simulink model "ofdm_txrx_supermimo_coded"  (Simulink 7.7)
settings: Solver=VariableStepDiscrete  SolverMode=SingleTasking  StartTime=0.0  StopTime=2*simOnly_simLength  RelTol=1e-3
PostLoadFcn:
  | ofdm_rx_supermimo_init
InitFcn:
  | ofdm_rx_supermimo_init
StartFcn:
  | ofdm_rx_supermimo_init
values from neighbouring files (symbols this model uses):
  ActionID_AFTransmit = 31    [ofdm_tx_supermimo_init.m]
  ActionID_Disabled = 0    [ofdm_tx_supermimo_init.m]
  ActionID_SetFlagA = 62    [ofdm_tx_supermimo_init.m]
  ActionID_SetFlagB = 61    [ofdm_tx_supermimo_init.m]
  BER_RAM_init_size = 2048    [ofdm_tx_supermimo_init.m]
  channel_h = [1 0; 1 0] * 0.3    [ofdm_tx_supermimo_init.m]
  CPLength = 16    [ofdm_tx_supermimo_init.m]
  longCorr_coef_i = [-4*real(LTS) 0]    [ofdm_rx_supermimo_init.m]
  longCorr_coef_q = [4*imag(LTS) 0]    [ofdm_rx_supermimo_init.m]
  max_CP_length = 16    [ofdm_tx_supermimo_init.m]
  max_num_baseRateSymbols = 31    [ofdm_tx_supermimo_init.m]
  max_num_subcarriers = 64    [ofdm_tx_supermimo_init.m]
  max_num_trainingSymbols = 15    [ofdm_tx_supermimo_init.m]
  max_numBytes = 16384    [ofdm_tx_supermimo_init.m]
  max_OFDM_symbols = 1023    [ofdm_tx_supermimo_init.m]
  modConstellation_qam16 = .75*[1 3 -1 -3]./3    [ofdm_tx_supermimo_init.m]
  modConstellation_qam64 = 0.875*[3 1 5 7 -3 -1 -5 -7]./7    [ofdm_tx_supermimo_init.m]
  modConstellation_qpsk = [1 -1]./sqrt(2)    [ofdm_tx_supermimo_init.m]
  numBaseRateSymbols = numBaseRateSymbols *2    [ofdm_tx_supermimo_init.m]
  numSubcarriers = 64    [ofdm_tx_supermimo_init.m]
  numTrainingSymbols = 2    [ofdm_tx_supermimo_init.m]
  pktTiming_count_payloadStart = pktTiming_count_lsStart + pktTiming_ls_num*pktTiming_ls_length    [ofdm_rx_supermimo_init.m]
  preamble = preamble_scale*[shortsyms_10 longsyms_2]    [ofdm_tx_supermimo_init.m]
  preamble_ROM = preamble_I_ROM + preamble_Q_ROM*2^16    [ofdm_tx_supermimo_init.m]
  RAM_init_size = max_numBytes    (= 16384)    [ofdm_tx_supermimo_init.m]
  RxDataScrambling_Seq = [40 198 78 63 82 173 102 245 48 111 172 115 147 230 216 93 72 65 62 2 205 242 122 90 128 83 105 97 73 10 5 252]    [ofdm_tx_supermimo_init.m]
  shortSymbol_time = shortSymbol_time(1:16).'    [ofdm_tx_supermimo_init.m]
  simOnly_numSamples = length(preamble)+( (numSubcarriers+CPLength)*(numTrainingSymbols + numBaseRateSymbols + numFullRateSymbols/2) )    [ofdm_tx_supermimo_init.m]
  train = [train train]    [ofdm_tx_supermimo_init.m]
  TxDataScrambling_Seq = [40 198 78 63 82 173 102 245 48 111 172 115 147 230 216 93 72 65 62 2 205 242 122 90 128 83 105 97 73 10 5 252]    [ofdm_tx_supermimo_init.m]
undefined symbols (not defined in the file or in its folder): AntA, AntB, BaseRate, Down, gcbh, numBuffs, numBytes, Up, xlBlockLoadCallback, xlGetNormalizedPeriod, xlipgetpartsetting
libraries: xbsIndex_r4 x5430
(part 2 of 49)

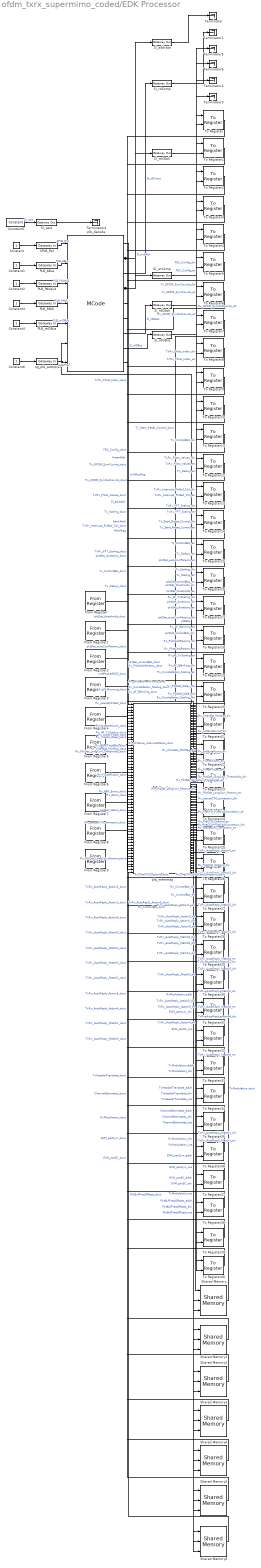
== system ofdm_txrx_supermimo_coded/EDK Processor ==
  Constant{,1..4,6} (6 alike) : Constant
  Constant5 : xbsIndex_r4/Constant  const=0  gui_display_data_type=Fixed-point  arith_type=Unsigned  n_bits=1  bin_pt=0  preci_type=Single  exp_width=8  frac_width=24  explicit_period=on  period=xlGetNormalizedPeriod()  equ=P=C  opselect=C  inp2=PCIN>>17  opr=+  inp1=P  carry=CIN  dbl_ovrd=off  block_type=constant
  From Register : xbsIndex_r4/From Register  shared_memory_name='midPacketRSSI'  init=0  period=xlGetNormalizedPeriod()  ownership=Owned and initialized elsewhere  gui_display_data_type=Fixed-point  arith_type=Unsigned  n_bits=32  bin_pt=0  preci_type=Single  dbl_ovrd=off  block_type=fromreg
      settings: AttributesFormatString=<< %<shared_memory_name> >>  infoedit="Register block that reads data to a shared memory register.  Delay of one sample period."
  From Register1 : xbsIndex_r4/From Register  shared_memory_name='Tx_PktRunning'  init=0  period=xlGetNormalizedPeriod()  ownership=Owned and initialized elsewhere  gui_display_data_type=Fixed-point  arith_type=Unsigned  n_bits=32  bin_pt=0  preci_type=Single  dbl_ovrd=off  block_type=fromreg
      settings: AttributesFormatString=<< %<shared_memory_name> >>  infoedit="Register block that reads data to a shared memory register.  Delay of one sample period."
  From Register2 : xbsIndex_r4/From Register  shared_memory_name='Rx_pktDetEventCount'  init=0  period=xlGetNormalizedPeriod()  ownership=Owned and initialized elsewhere  gui_display_data_type=Fixed-point  arith_type=Unsigned  n_bits=32  bin_pt=0  preci_type=Single  dbl_ovrd=off  block_type=fromreg
      settings: AttributesFormatString=<< %<shared_memory_name> >>  infoedit="Register block that reads data to a shared memory register.  Delay of one sample period."
  From Register3 : xbsIndex_r4/From Register  shared_memory_name='Rx_coarseCFOest'  init=0  period=xlGetNormalizedPeriod()  ownership=Owned and initialized elsewhere  gui_display_data_type=Fixed-point  arith_type=Unsigned  n_bits=32  bin_pt=0  preci_type=Single  dbl_ovrd=off  block_type=fromreg
      settings: AttributesFormatString=<< %<shared_memory_name> >>  infoedit="Register block that reads data to a shared memory register.  Delay of one sample period."
  From Register4 : xbsIndex_r4/From Register  shared_memory_name='Rx_pilotCFOest'  init=0  period=xlGetNormalizedPeriod()  ownership=Owned and initialized elsewhere  gui_display_data_type=Fixed-point  arith_type=Unsigned  n_bits=32  bin_pt=0  preci_type=Single  dbl_ovrd=off  block_type=fromreg
      settings: AttributesFormatString=<< %<shared_memory_name> >>  infoedit="Register block that reads data to a shared memory register.  Delay of one sample period."
  From Register5 : xbsIndex_r4/From Register  shared_memory_name='Rx_pktDone_interruptStatus'  init=0  period=xlGetNormalizedPeriod()  ownership=Owned and initialized elsewhere  gui_display_data_type=Fixed-point  arith_type=Unsigned  n_bits=32  bin_pt=0  preci_type=Single  dbl_ovrd=off  block_type=fromreg
      settings: AttributesFormatString=<< %<shared_memory_name> >>  infoedit="Register block that reads data to a shared memory register.  Delay of one sample period."
  From Register6 : xbsIndex_r4/From Register  shared_memory_name='Rx_BER_TotalBits'  init=0  period=xlGetNormalizedPeriod()  ownership=Owned and initialized elsewhere  gui_display_data_type=Fixed-point  arith_type=Unsigned  n_bits=32  bin_pt=0  preci_type=Single  dbl_ovrd=off  block_type=fromreg
      settings: AttributesFormatString=<< %<shared_memory_name> >>  infoedit="Register block that reads data to a shared memory register.  Delay of one sample period."
  From Register7 : xbsIndex_r4/From Register  shared_memory_name='Rx_BER_Errors'  init=0  period=xlGetNormalizedPeriod()  ownership=Owned and initialized elsewhere  gui_display_data_type=Fixed-point  arith_type=Unsigned  n_bits=32  bin_pt=0  preci_type=Single  dbl_ovrd=off  block_type=fromreg
      settings: AttributesFormatString=<< %<shared_memory_name> >>  infoedit="Register block that reads data to a shared memory register.  Delay of one sample period."
  From Register8 : xbsIndex_r4/From Register  shared_memory_name='Rx_Gains'  init=0  period=xlGetNormalizedPeriod()  ownership=Owned and initialized elsewhere  gui_display_data_type=Fixed-point  arith_type=Unsigned  n_bits=32  bin_pt=0  preci_type=Single  dbl_ovrd=off  block_type=fromreg
      settings: AttributesFormatString=<< %<shared_memory_name> >>  infoedit="Register block that reads data to a shared memory register.  Delay of one sample period."
  From Register9 : xbsIndex_r4/From Register  shared_memory_name='pktDet_status'  init=0  period=xlGetNormalizedPeriod()  ownership=Owned and initialized elsewhere  gui_display_data_type=Fixed-point  arith_type=Unsigned  n_bits=14  bin_pt=0  preci_type=Single  dbl_ovrd=off  block_type=fromreg
      settings: AttributesFormatString=<< %<shared_memory_name> >>  infoedit="Register block that reads data to a shared memory register.  Delay of one sample period."
  PLB_ABus : xbsIndex_r4/Gateway In  gui_display_data_type=Fixed-point  arith_type=Unsigned  n_bits=32  bin_pt=0  preci_type=Single  exp_width=8  frac_width=24  quantization="Round  (unbiased: +/- Inf)"  overflow=Saturate  period=xlGetNormalizedPeriod()  dbl_ovrd=off  timing_constraint=None  locs_specified=off  LOCs={}  inherit_from_input=off  UseAsADC=off  ADCChannel='1'  hdl_port=on  block_type=gatewayin
      settings: infoedit="Gateway in block.  Converts inputs of type Simulink integer, single, double and fixed point to  Xilinx fixed-point or floating-point data type.<br><br>Hardware notes:  In hardware these blocks become top level input ports."  sginterface={'Xilinx'=>{'jtaghwcosim'=>{'non_memory_mapped_port'=>'PLB_ABus'}},'iopad'=>{'constraint'=>'#'}}
  PLB_PAValid : xbsIndex_r4/Gateway In  gui_display_data_type=Fixed-point  arith_type=Unsigned  n_bits=1  bin_pt=0  preci_type=Single  exp_width=8  frac_width=24  quantization="Round  (unbiased: +/- Inf)"  overflow=Saturate  period=xlGetNormalizedPeriod()  dbl_ovrd=off  timing_constraint=None  locs_specified=off  LOCs={}  inherit_from_input=off  UseAsADC=off  ADCChannel='1'  hdl_port=on  block_type=gatewayin
      settings: infoedit="Gateway in block.  Converts inputs of type Simulink integer, single, double and fixed point to  Xilinx fixed-point or floating-point data type.<br><br>Hardware notes:  In hardware these blocks become top level input ports."  sginterface={'Xilinx'=>{'jtaghwcosim'=>{'non_memory_mapped_port'=>'PLB_PAValid'}},'iopad'=>{'constraint'=>'#'}}
  PLB_RNW : xbsIndex_r4/Gateway In  gui_display_data_type=Fixed-point  arith_type=Unsigned  n_bits=1  bin_pt=0  preci_type=Single  exp_width=8  frac_width=24  quantization="Round  (unbiased: +/- Inf)"  overflow=Saturate  period=xlGetNormalizedPeriod()  dbl_ovrd=off  timing_constraint=None  locs_specified=off  LOCs={}  inherit_from_input=off  UseAsADC=off  ADCChannel='1'  hdl_port=on  block_type=gatewayin
      settings: infoedit="Gateway in block.  Converts inputs of type Simulink integer, single, double and fixed point to  Xilinx fixed-point or floating-point data type.<br><br>Hardware notes:  In hardware these blocks become top level input ports."  sginterface={'Xilinx'=>{'jtaghwcosim'=>{'non_memory_mapped_port'=>'PLB_RNW'}},'iopad'=>{'constraint'=>'#'}}
  PLB_wrDBus : xbsIndex_r4/Gateway In  gui_display_data_type=Fixed-point  arith_type=Unsigned  n_bits=32  bin_pt=0  preci_type=Single  exp_width=8  frac_width=24  quantization="Round  (unbiased: +/- Inf)"  overflow=Saturate  period=xlGetNormalizedPeriod()  dbl_ovrd=off  timing_constraint=None  locs_specified=off  LOCs={}  inherit_from_input=off  UseAsADC=off  ADCChannel='1'  hdl_port=on  block_type=gatewayin
      settings: infoedit="Gateway in block.  Converts inputs of type Simulink integer, single, double and fixed point to  Xilinx fixed-point or floating-point data type.<br><br>Hardware notes:  In hardware these blocks become top level input ports."  sginterface={'Xilinx'=>{'jtaghwcosim'=>{'non_memory_mapped_port'=>'PLB_wrDBus'}},'iopad'=>{'constraint'=>'#'}}
  SPLB_Rst : xbsIndex_r4/Gateway In  gui_display_data_type=Fixed-point  arith_type=Unsigned  n_bits=1  bin_pt=0  preci_type=Single  exp_width=8  frac_width=24  quantization="Round  (unbiased: +/- Inf)"  overflow=Saturate  period=xlGetNormalizedPeriod()  dbl_ovrd=off  timing_constraint=None  locs_specified=off  LOCs={}  inherit_from_input=off  UseAsADC=off  ADCChannel='1'  hdl_port=on  block_type=gatewayin
      settings: infoedit="Gateway in block.  Converts inputs of type Simulink integer, single, double and fixed point to  Xilinx fixed-point or floating-point data type.<br><br>Hardware notes:  In hardware these blocks become top level input ports."  sginterface={'Xilinx'=>{'jtaghwcosim'=>{'non_memory_mapped_port'=>'SPLB_Rst'}},'iopad'=>{'constraint'=>'#'}}
  Shared Memory : xbsIndex_r4/Shared Memory  shared_memory_name='TxModulation'  depth=192  ownership=Owned and Initialized Elsewhere  initVector=sin(pi*(0:15)/16)  en=off  mutex=Unprotected  mode=Read and Write  write_mode=Read Before Write  time_out=0  latency=1  explicit_data_type=off  gui_display_data_type=Fixed-point  arith_type=Unsigned  n_bits=4  bin_pt=0  preci_type=Single  implementation=Block RAM  use_rpm=off  block_type=shmem
      settings: AttributesFormatString=<< %<shared_memory_name> >>
  Shared Memory1 : xbsIndex_r4/Shared Memory  shared_memory_name='TxHeaderTranslate'  depth=1024  ownership=Owned and Initialized Elsewhere  initVector=sin(pi*(0:15)/16)  en=off  mutex=Unprotected  mode=Read and Write  write_mode=Read Before Write  time_out=0  latency=1  explicit_data_type=off  gui_display_data_type=Fixed-point  arith_type=Unsigned  n_bits=10  bin_pt=0  preci_type=Single  implementation=Block RAM  use_rpm=off  block_type=shmem
      settings: AttributesFormatString=<< %<shared_memory_name> >>
  Shared Memory2 : xbsIndex_r4/Shared Memory  shared_memory_name='ChannelEstimates'  depth=256  ownership=Owned and Initialized Elsewhere  initVector=sin(pi*(0:15)/16)  en=off  mutex=Unprotected  mode=Read and Write  write_mode=Read Before Write  time_out=0  latency=1  explicit_data_type=off  gui_display_data_type=Fixed-point  arith_type=Unsigned  n_bits=32  bin_pt=0  preci_type=Single  implementation=Block RAM  use_rpm=off  block_type=shmem
      settings: AttributesFormatString=<< %<shared_memory_name> >>
  Shared Memory3 : xbsIndex_r4/Shared Memory  shared_memory_name='RxModulation'  depth=192  ownership=Owned and Initialized Elsewhere  initVector=sin(pi*(0:15)/16)  en=off  mutex=Unprotected  mode=Read and Write  write_mode=Read Before Write  time_out=0  latency=1  explicit_data_type=off  gui_display_data_type=Fixed-point  arith_type=Unsigned  n_bits=4  bin_pt=0  preci_type=Single  implementation=Block RAM  use_rpm=off  block_type=shmem
      settings: AttributesFormatString=<< %<shared_memory_name> >>
  Shared Memory4 : xbsIndex_r4/Shared Memory  shared_memory_name='EVM_perSym'  depth=256  ownership=Owned and Initialized Elsewhere  initVector=sin(pi*(0:15)/16)  en=off  mutex=Unprotected  mode=Read and Write  write_mode=Read Before Write  time_out=0  latency=1  explicit_data_type=off  gui_display_data_type=Fixed-point  arith_type="Signed  (2's comp)"  n_bits=32  bin_pt=14  preci_type=Single  implementation=Block RAM  use_rpm=off  block_type=shmem
      settings: AttributesFormatString=<< %<shared_memory_name> >>
  Shared Memory5 : xbsIndex_r4/Shared Memory  shared_memory_name='EVM_perSC'  depth=64  ownership=Owned and Initialized Elsewhere  initVector=sin(pi*(0:15)/16)  en=off  mutex=Unprotected  mode=Read and Write  write_mode=Read Before Write  time_out=0  latency=1  explicit_data_type=off  gui_display_data_type=Fixed-point  arith_type="Signed  (2's comp)"  n_bits=32  bin_pt=22  preci_type=Single  implementation=Block RAM  use_rpm=off  block_type=shmem
      settings: AttributesFormatString=<< %<shared_memory_name> >>
  Shared Memory6 : xbsIndex_r4/Shared Memory  shared_memory_name='PktBufFreqOffsets'  depth=32  ownership=Owned and Initialized Elsewhere  initVector=sin(pi*(0:15)/16)  en=off  mutex=Unprotected  mode=Read and Write  write_mode=Read Before Write  time_out=0  latency=1  explicit_data_type=off  gui_display_data_type=Fixed-point  arith_type=Unsigned  n_bits=32  bin_pt=32  preci_type=Single  implementation=Block RAM  use_rpm=off  block_type=shmem
      settings: AttributesFormatString=<< %<shared_memory_name> >>
  Sl_addrAck : xbsIndex_r4/Gateway Out  inherit_from_input=off  hdl_port=on  timing_constraint=None  locs_specified=off  LOCs={}  UseAsDAC=off  DACChannel='1'  block_type=gatewayout
      settings: infoedit="Gateway out block.  Converts Xilinx fixed-point or floating-point type inputs into ouputs of type Simulink integer, single, double, or fixed point.<br><br>Hardware notes:  In hardware these blocks become top level output ports or are discarded, depending on how they are configured."  sginterface={'Xilinx'=>{'jtaghwcosim'=>{'non_memory_mapped_port'=>'Sl_addrAck'}},'iopad'=>{'constraint'=>'#'}}
  Sl_rdComp : xbsIndex_r4/Gateway Out  inherit_from_input=off  hdl_port=on  timing_constraint=None  locs_specified=off  LOCs={}  UseAsDAC=off  DACChannel='1'  block_type=gatewayout
      settings: infoedit="Gateway out block.  Converts Xilinx fixed-point or floating-point type inputs into ouputs of type Simulink integer, single, double, or fixed point.<br><br>Hardware notes:  In hardware these blocks become top level output ports or are discarded, depending on how they are configured."  sginterface={'Xilinx'=>{'jtaghwcosim'=>{'non_memory_mapped_port'=>'Sl_rdComp'}},'iopad'=>{'constraint'=>'#'}}
  Sl_rdDAck : xbsIndex_r4/Gateway Out  inherit_from_input=off  hdl_port=on  timing_constraint=None  locs_specified=off  LOCs={}  UseAsDAC=off  DACChannel='1'  block_type=gatewayout
      settings: infoedit="Gateway out block.  Converts Xilinx fixed-point or floating-point type inputs into ouputs of type Simulink integer, single, double, or fixed point.<br><br>Hardware notes:  In hardware these blocks become top level output ports or are discarded, depending on how they are configured."  sginterface={'Xilinx'=>{'jtaghwcosim'=>{'non_memory_mapped_port'=>'Sl_rdDAck'}},'iopad'=>{'constraint'=>'#'}}
  Sl_rdDBus : xbsIndex_r4/Gateway Out  inherit_from_input=off  hdl_port=on  timing_constraint=None  locs_specified=off  LOCs={}  UseAsDAC=off  DACChannel='1'  block_type=gatewayout
      settings: infoedit="Gateway out block.  Converts Xilinx fixed-point or floating-point type inputs into ouputs of type Simulink integer, single, double, or fixed point.<br><br>Hardware notes:  In hardware these blocks become top level output ports or are discarded, depending on how they are configured."  sginterface={'Xilinx'=>{'jtaghwcosim'=>{'non_memory_mapped_port'=>'Sl_rdDBus'}},'iopad'=>{'constraint'=>'#'}}
  Sl_wait : xbsIndex_r4/Gateway Out  inherit_from_input=off  hdl_port=on  timing_constraint=None  locs_specified=off  LOCs={}  UseAsDAC=off  DACChannel='1'  block_type=gatewayout
      settings: infoedit="Gateway out block.  Converts Xilinx fixed-point or floating-point type inputs into ouputs of type Simulink integer, single, double, or fixed point.<br><br>Hardware notes:  In hardware these blocks become top level output ports or are discarded, depending on how they are configured."  sginterface={'Xilinx'=>{'jtaghwcosim'=>{'non_memory_mapped_port'=>'Sl_wait'}},'iopad'=>{'constraint'=>'#'}}
  Sl_wrComp : xbsIndex_r4/Gateway Out  inherit_from_input=off  hdl_port=on  timing_constraint=None  locs_specified=off  LOCs={}  UseAsDAC=off  DACChannel='1'  block_type=gatewayout
      settings: infoedit="Gateway out block.  Converts Xilinx fixed-point or floating-point type inputs into ouputs of type Simulink integer, single, double, or fixed point.<br><br>Hardware notes:  In hardware these blocks become top level output ports or are discarded, depending on how they are configured."  sginterface={'Xilinx'=>{'jtaghwcosim'=>{'non_memory_mapped_port'=>'Sl_wrComp'}},'iopad'=>{'constraint'=>'#'}}
  Sl_wrDAck : xbsIndex_r4/Gateway Out  inherit_from_input=off  hdl_port=on  timing_constraint=None  locs_specified=off  LOCs={}  UseAsDAC=off  DACChannel='1'  block_type=gatewayout
      settings: infoedit="Gateway out block.  Converts Xilinx fixed-point or floating-point type inputs into ouputs of type Simulink integer, single, double, or fixed point.<br><br>Hardware notes:  In hardware these blocks become top level output ports or are discarded, depending on how they are configured."  sginterface={'Xilinx'=>{'jtaghwcosim'=>{'non_memory_mapped_port'=>'Sl_wrDAck'}},'iopad'=>{'constraint'=>'#'}}
  Terminator{,1..6} (7 alike) : Terminator
  To Register : xbsIndex_r4/To Register  shared_memory_name='FEC_Config'  init=0  ownership=Owned and initialized elsewhere  explicit_data_type=on  gui_display_data_type=Fixed-point  arith_type=Unsigned  n_bits=32  bin_pt=0  preci_type=Single  dbl_ovrd=off  block_type=toreg
      settings: AttributesFormatString=<< %<shared_memory_name> >>  infoedit="Register block that writes data to a shared memory register.  Delay of one sample period."
  To Register1 : xbsIndex_r4/To Register  shared_memory_name='Tx_OFDM_SymCounts'  init=0  ownership=Owned and initialized elsewhere  explicit_data_type=on  gui_display_data_type=Fixed-point  arith_type=Unsigned  n_bits=32  bin_pt=0  preci_type=Single  dbl_ovrd=off  block_type=toreg
      settings: AttributesFormatString=<< %<shared_memory_name> >>  infoedit="Register block that writes data to a shared memory register.  Delay of one sample period."
  To Register10 : xbsIndex_r4/To Register  shared_memory_name='Tx_Scaling'  init=0  ownership=Owned and initialized elsewhere  explicit_data_type=on  gui_display_data_type=Fixed-point  arith_type=Unsigned  n_bits=32  bin_pt=0  preci_type=Single  dbl_ovrd=off  block_type=toreg
      settings: AttributesFormatString=<< %<shared_memory_name> >>  infoedit="Register block that writes data to a shared memory register.  Delay of one sample period."
  To Register11 : xbsIndex_r4/To Register  shared_memory_name='pktDet_thresholds'  init=0  ownership=Owned and initialized elsewhere  explicit_data_type=on  gui_display_data_type=Fixed-point  arith_type=Unsigned  n_bits=32  bin_pt=0  preci_type=Single  dbl_ovrd=off  block_type=toreg
      settings: AttributesFormatString=<< %<shared_memory_name> >>  infoedit="Register block that writes data to a shared memory register.  Delay of one sample period."
  To Register12 : xbsIndex_r4/To Register  shared_memory_name='pktDet_durations'  init=0  ownership=Owned and initialized elsewhere  explicit_data_type=on  gui_display_data_type=Fixed-point  arith_type=Unsigned  n_bits=32  bin_pt=0  preci_type=Single  dbl_ovrd=off  block_type=toreg
      settings: AttributesFormatString=<< %<shared_memory_name> >>  infoedit="Register block that writes data to a shared memory register.  Delay of one sample period."
  To Register13 : xbsIndex_r4/To Register  shared_memory_name='pktDet_autoCorrParams'  init=0  ownership=Owned and initialized elsewhere  explicit_data_type=on  gui_display_data_type=Fixed-point  arith_type=Unsigned  n_bits=32  bin_pt=0  preci_type=Single  dbl_ovrd=off  block_type=toreg
      settings: AttributesFormatString=<< %<shared_memory_name> >>  infoedit="Register block that writes data to a shared memory register.  Delay of one sample period."
  To Register14 : xbsIndex_r4/To Register  shared_memory_name='pktDet_controlBits'  init=0  ownership=Owned and initialized elsewhere  explicit_data_type=on  gui_display_data_type=Fixed-point  arith_type=Unsigned  n_bits=32  bin_pt=0  preci_type=Single  dbl_ovrd=off  block_type=toreg
      settings: AttributesFormatString=<< %<shared_memory_name> >>  infoedit="Register block that writes data to a shared memory register.  Delay of one sample period."
  To Register15 : xbsIndex_r4/To Register  shared_memory_name='Rx_AF_TxScaling'  init=0  ownership=Owned and initialized elsewhere  explicit_data_type=on  gui_display_data_type=Fixed-point  arith_type=Unsigned  n_bits=32  bin_pt=0  preci_type=Single  dbl_ovrd=off  block_type=toreg
      settings: AttributesFormatString=<< %<shared_memory_name> >>  infoedit="Register block that writes data to a shared memory register.  Delay of one sample period."
  To Register16 : xbsIndex_r4/To Register  shared_memory_name='Rx_AF_Blanking'  init=0  ownership=Owned and initialized elsewhere  explicit_data_type=on  gui_display_data_type=Fixed-point  arith_type=Unsigned  n_bits=32  bin_pt=0  preci_type=Single  dbl_ovrd=off  block_type=toreg
      settings: AttributesFormatString=<< %<shared_memory_name> >>  infoedit="Register block that writes data to a shared memory register.  Delay of one sample period."
  To Register17 : xbsIndex_r4/To Register  shared_memory_name='Rx_PilotCalcParams'  init=0  ownership=Owned and initialized elsewhere  explicit_data_type=on  gui_display_data_type=Fixed-point  arith_type=Unsigned  n_bits=32  bin_pt=0  preci_type=Single  dbl_ovrd=off  block_type=toreg
      settings: AttributesFormatString=<< %<shared_memory_name> >>  infoedit="Register block that writes data to a shared memory register.  Delay of one sample period."
  To Register18 : xbsIndex_r4/To Register  shared_memory_name='Rx_Constellation_Scaling'  init=0  ownership=Owned and initialized elsewhere  explicit_data_type=on  gui_display_data_type=Fixed-point  arith_type=Unsigned  n_bits=32  bin_pt=0  preci_type=Single  dbl_ovrd=off  block_type=toreg
      settings: AttributesFormatString=<< %<shared_memory_name> >>  infoedit="Register block that writes data to a shared memory register.  Delay of one sample period."
  To Register19 : xbsIndex_r4/To Register  shared_memory_name='Rx_PktDet_Delay'  init=0  ownership=Owned and initialized elsewhere  explicit_data_type=on  gui_display_data_type=Fixed-point  arith_type=Unsigned  n_bits=32  bin_pt=0  preci_type=Single  dbl_ovrd=off  block_type=toreg
      settings: AttributesFormatString=<< %<shared_memory_name> >>  infoedit="Register block that writes data to a shared memory register.  Delay of one sample period."
  To Register2 : xbsIndex_r4/To Register  shared_memory_name='Rx_OFDM_SymbolCounts'  init=0  ownership=Owned and initialized elsewhere  explicit_data_type=on  gui_display_data_type=Fixed-point  arith_type=Unsigned  n_bits=32  bin_pt=0  preci_type=Single  dbl_ovrd=off  block_type=toreg
      settings: AttributesFormatString=<< %<shared_memory_name> >>  infoedit="Register block that writes data to a shared memory register.  Delay of one sample period."
  To Register20 : xbsIndex_r4/To Register  shared_memory_name='Rx_ChanEst_MinMag'  init=0  ownership=Owned and initialized elsewhere  explicit_data_type=on  gui_display_data_type=Fixed-point  arith_type=Unsigned  n_bits=32  bin_pt=0  preci_type=Single  dbl_ovrd=off  block_type=toreg
      settings: AttributesFormatString=<< %<shared_memory_name> >>  infoedit="Register block that writes data to a shared memory register.  Delay of one sample period."
  To Register21 : xbsIndex_r4/To Register  shared_memory_name='Rx_pktByteNums'  init=0  ownership=Owned and initialized elsewhere  explicit_data_type=on  gui_display_data_type=Fixed-point  arith_type=Unsigned  n_bits=32  bin_pt=0  preci_type=Single  dbl_ovrd=off  block_type=toreg
      settings: AttributesFormatString=<< %<shared_memory_name> >>  infoedit="Register block that writes data to a shared memory register.  Delay of one sample period."
  To Register22 : xbsIndex_r4/To Register  shared_memory_name='Rx_FixedPktLen'  init=0  ownership=Owned and initialized elsewhere  explicit_data_type=on  gui_display_data_type=Fixed-point  arith_type=Unsigned  n_bits=32  bin_pt=0  preci_type=Single  dbl_ovrd=off  block_type=toreg
      settings: AttributesFormatString=<< %<shared_memory_name> >>  infoedit="Register block that writes data to a shared memory register.  Delay of one sample period."
  To Register23 : xbsIndex_r4/To Register  shared_memory_name='Rx_PktDet_LongCorr_Thresholds'  init=0  ownership=Owned and initialized elsewhere  explicit_data_type=on  gui_display_data_type=Fixed-point  arith_type=Unsigned  n_bits=32  bin_pt=0  preci_type=Single  dbl_ovrd=off  block_type=toreg
      settings: AttributesFormatString=<< %<shared_memory_name> >>  infoedit="Register block that writes data to a shared memory register.  Delay of one sample period."
  To Register24 : xbsIndex_r4/To Register  shared_memory_name='Rx_PktDet_LongCorr_Params'  init=0  ownership=Owned and initialized elsewhere  explicit_data_type=on  gui_display_data_type=Fixed-point  arith_type=Unsigned  n_bits=32  bin_pt=0  preci_type=Single  dbl_ovrd=off  block_type=toreg
      settings: AttributesFormatString=<< %<shared_memory_name> >>  infoedit="Register block that writes data to a shared memory register.  Delay of one sample period."
  To Register25 : xbsIndex_r4/To Register  shared_memory_name='Rx_coarseCFO_correction'  init=0  ownership=Owned and initialized elsewhere  explicit_data_type=on  gui_display_data_type=Fixed-point  arith_type=Unsigned  n_bits=32  bin_pt=0  preci_type=Single  dbl_ovrd=off  block_type=toreg
      settings: AttributesFormatString=<< %<shared_memory_name> >>  infoedit="Register block that writes data to a shared memory register.  Delay of one sample period."
  To Register26 : xbsIndex_r4/To Register  shared_memory_name='Rx_PreCFO_PilotCalcCorrection'  init=0  ownership=Owned and initialized elsewhere  explicit_data_type=on  gui_display_data_type=Fixed-point  arith_type=Unsigned  n_bits=32  bin_pt=0  preci_type=Single  dbl_ovrd=off  block_type=toreg
      settings: AttributesFormatString=<< %<shared_memory_name> >>  infoedit="Register block that writes data to a shared memory register.  Delay of one sample period."
  To Register27 : xbsIndex_r4/To Register  shared_memory_name='Rx_PreCFO_Options'  init=0  ownership=Owned and initialized elsewhere  explicit_data_type=on  gui_display_data_type=Fixed-point  arith_type=Unsigned  n_bits=32  bin_pt=0  preci_type=Single  dbl_ovrd=off  block_type=toreg
      settings: AttributesFormatString=<< %<shared_memory_name> >>  infoedit="Register block that writes data to a shared memory register.  Delay of one sample period."
  To Register28 : xbsIndex_r4/To Register  shared_memory_name='Rx_ControlBits'  init=0  ownership=Owned and initialized elsewhere  explicit_data_type=on  gui_display_data_type=Fixed-point  arith_type=Unsigned  n_bits=32  bin_pt=0  preci_type=Single  dbl_ovrd=off  block_type=toreg
      settings: AttributesFormatString=<< %<shared_memory_name> >>  infoedit="Register block that writes data to a shared memory register.  Delay of one sample period."
  To Register29 : xbsIndex_r4/To Register  shared_memory_name='TxRx_AutoReply_Action3'  init=0  ownership=Owned and initialized elsewhere  explicit_data_type=on  gui_display_data_type=Fixed-point  arith_type=Unsigned  n_bits=32  bin_pt=0  preci_type=Single  dbl_ovrd=off  block_type=toreg
      settings: AttributesFormatString=<< %<shared_memory_name> >>  infoedit="Register block that writes data to a shared memory register.  Delay of one sample period."
  To Register3 : xbsIndex_r4/To Register  shared_memory_name='TxRx_Pilots_Values'  init=0  ownership=Owned and initialized elsewhere  explicit_data_type=on  gui_display_data_type=Fixed-point  arith_type=Unsigned  n_bits=32  bin_pt=0  preci_type=Single  dbl_ovrd=off  block_type=toreg
      settings: AttributesFormatString=<< %<shared_memory_name> >>  infoedit="Register block that writes data to a shared memory register.  Delay of one sample period."
  To Register30 : xbsIndex_r4/To Register  shared_memory_name='TxRx_AutoReply_Action2'  init=0  ownership=Owned and initialized elsewhere  explicit_data_type=on  gui_display_data_type=Fixed-point  arith_type=Unsigned  n_bits=32  bin_pt=0  preci_type=Single  dbl_ovrd=off  block_type=toreg
      settings: AttributesFormatString=<< %<shared_memory_name> >>  infoedit="Register block that writes data to a shared memory register.  Delay of one sample period."
  To Register31 : xbsIndex_r4/To Register  shared_memory_name='TxRx_AutoReply_Action1'  init=0  ownership=Owned and initialized elsewhere  explicit_data_type=on  gui_display_data_type=Fixed-point  arith_type=Unsigned  n_bits=32  bin_pt=0  preci_type=Single  dbl_ovrd=off  block_type=toreg
      settings: AttributesFormatString=<< %<shared_memory_name> >>  infoedit="Register block that writes data to a shared memory register.  Delay of one sample period."
  To Register32 : xbsIndex_r4/To Register  shared_memory_name='TxRx_AutoReply_Action0'  init=0  ownership=Owned and initialized elsewhere  explicit_data_type=on  gui_display_data_type=Fixed-point  arith_type=Unsigned  n_bits=32  bin_pt=0  preci_type=Single  dbl_ovrd=off  block_type=toreg
      settings: AttributesFormatString=<< %<shared_memory_name> >>  infoedit="Register block that writes data to a shared memory register.  Delay of one sample period."
  To Register33 : xbsIndex_r4/To Register  shared_memory_name='TxRx_AutoReply_Match5'  init=0  ownership=Owned and initialized elsewhere  explicit_data_type=on  gui_display_data_type=Fixed-point  arith_type=Unsigned  n_bits=32  bin_pt=0  preci_type=Single  dbl_ovrd=off  block_type=toreg
      settings: AttributesFormatString=<< %<shared_memory_name> >>  infoedit="Register block that writes data to a shared memory register.  Delay of one sample period."
  To Register34 : xbsIndex_r4/To Register  shared_memory_name='TxRx_AutoReply_Match4'  init=0  ownership=Owned and initialized elsewhere  explicit_data_type=on  gui_display_data_type=Fixed-point  arith_type=Unsigned  n_bits=32  bin_pt=0  preci_type=Single  dbl_ovrd=off  block_type=toreg
      settings: AttributesFormatString=<< %<shared_memory_name> >>  infoedit="Register block that writes data to a shared memory register.  Delay of one sample period."
  To Register35 : xbsIndex_r4/To Register  shared_memory_name='TxRx_AutoReply_Match3'  init=0  ownership=Owned and initialized elsewhere  explicit_data_type=on  gui_display_data_type=Fixed-point  arith_type=Unsigned  n_bits=32  bin_pt=0  preci_type=Single  dbl_ovrd=off  block_type=toreg
      settings: AttributesFormatString=<< %<shared_memory_name> >>  infoedit="Register block that writes data to a shared memory register.  Delay of one sample period."
  To Register36 : xbsIndex_r4/To Register  shared_memory_name='TxRx_AutoReply_Match2'  init=0  ownership=Owned and initialized elsewhere  explicit_data_type=on  gui_display_data_type=Fixed-point  arith_type=Unsigned  n_bits=32  bin_pt=0  preci_type=Single  dbl_ovrd=off  block_type=toreg
      settings: AttributesFormatString=<< %<shared_memory_name> >>  infoedit="Register block that writes data to a shared memory register.  Delay of one sample period."
  To Register37 : xbsIndex_r4/To Register  shared_memory_name='TxRx_AutoReply_Action5'  init=0  ownership=Owned and initialized elsewhere  explicit_data_type=on  gui_display_data_type=Fixed-point  arith_type=Unsigned  n_bits=32  bin_pt=0  preci_type=Single  dbl_ovrd=off  block_type=toreg
      settings: AttributesFormatString=<< %<shared_memory_name> >>  infoedit="Register block that writes data to a shared memory register.  Delay of one sample period."
  To Register38 : xbsIndex_r4/To Register  shared_memory_name='TxRx_AutoReply_Action4'  init=0  ownership=Owned and initialized elsewhere  explicit_data_type=on  gui_display_data_type=Fixed-point  arith_type=Unsigned  n_bits=32  bin_pt=0  preci_type=Single  dbl_ovrd=off  block_type=toreg
      settings: AttributesFormatString=<< %<shared_memory_name> >>  infoedit="Register block that writes data to a shared memory register.  Delay of one sample period."
  To Register39 : xbsIndex_r4/To Register  shared_memory_name='TxRx_AutoReply_Match1'  init=0  ownership=Owned and initialized elsewhere  explicit_data_type=on  gui_display_data_type=Fixed-point  arith_type=Unsigned  n_bits=32  bin_pt=0  preci_type=Single  dbl_ovrd=off  block_type=toreg
      settings: AttributesFormatString=<< %<shared_memory_name> >>  infoedit="Register block that writes data to a shared memory register.  Delay of one sample period."
  To Register4 : xbsIndex_r4/To Register  shared_memory_name='TxRx_Pilots_Index'  init=0  ownership=Owned and initialized elsewhere  explicit_data_type=on  gui_display_data_type=Fixed-point  arith_type=Unsigned  n_bits=32  bin_pt=0  preci_type=Single  dbl_ovrd=off  block_type=toreg
      settings: AttributesFormatString=<< %<shared_memory_name> >>  infoedit="Register block that writes data to a shared memory register.  Delay of one sample period."
  To Register40 : xbsIndex_r4/To Register  shared_memory_name='TxRx_AutoReply_Match0'  init=0  ownership=Owned and initialized elsewhere  explicit_data_type=on  gui_display_data_type=Fixed-point  arith_type=Unsigned  n_bits=32  bin_pt=0  preci_type=Single  dbl_ovrd=off  block_type=toreg
      settings: AttributesFormatString=<< %<shared_memory_name> >>  infoedit="Register block that writes data to a shared memory register.  Delay of one sample period."
  To Register5 : xbsIndex_r4/To Register  shared_memory_name='TxRx_Interrupt_PktBuf_Ctrl'  init=0  ownership=Owned and initialized elsewhere  explicit_data_type=on  gui_display_data_type=Fixed-point  arith_type=Unsigned  n_bits=32  bin_pt=0  preci_type=Single  dbl_ovrd=off  block_type=toreg
      settings: AttributesFormatString=<< %<shared_memory_name> >>  infoedit="Register block that writes data to a shared memory register.  Delay of one sample period."
  To Register6 : xbsIndex_r4/To Register  shared_memory_name='TxRx_FFT_Scaling'  init=0  ownership=Owned and initialized elsewhere  explicit_data_type=on  gui_display_data_type=Fixed-point  arith_type=Unsigned  n_bits=12  bin_pt=0  preci_type=Single  dbl_ovrd=off  block_type=toreg
      settings: AttributesFormatString=<< %<shared_memory_name> >>  infoedit="Register block that writes data to a shared memory register.  Delay of one sample period."
  To Register7 : xbsIndex_r4/To Register  shared_memory_name='Tx_Start_Reset_Control'  init=0  ownership=Owned and initialized elsewhere  explicit_data_type=on  gui_display_data_type=Fixed-point  arith_type=Unsigned  n_bits=32  bin_pt=0  preci_type=Single  dbl_ovrd=off  block_type=toreg
      settings: AttributesFormatString=<< %<shared_memory_name> >>  infoedit="Register block that writes data to a shared memory register.  Delay of one sample period."
  To Register8 : xbsIndex_r4/To Register  shared_memory_name='Tx_ControlBits'  init=0  ownership=Owned and initialized elsewhere  explicit_data_type=on  gui_display_data_type=Fixed-point  arith_type=Unsigned  n_bits=32  bin_pt=0  preci_type=Single  dbl_ovrd=off  block_type=toreg
      settings: AttributesFormatString=<< %<shared_memory_name> >>  infoedit="Register block that writes data to a shared memory register.  Delay of one sample period."
  To Register9 : xbsIndex_r4/To Register  shared_memory_name='Tx_Delays'  init=0  ownership=Owned and initialized elsewhere  explicit_data_type=on  gui_display_data_type=Fixed-point  arith_type=Unsigned  n_bits=32  bin_pt=0  preci_type=Single  dbl_ovrd=off  block_type=toreg
      settings: AttributesFormatString=<< %<shared_memory_name> >>  infoedit="Register block that writes data to a shared memory register.  Delay of one sample period."
  plb_decode : xbsIndex_r4/MCode  mfname=xlmax  explicit_period=off  period=1  dbl_ovrd=off  enable_stdout=off  enable_debug=off  mfilecontent=|  suppress_output=on  defparams={}  hide_port_list={}  block_type=mcode
      | function [wrDBusReg, addrAck, rdComp, wrDAck, bankAddr, RNWReg, rdDAck, rdDBus, linearAddr] = plb_bus_decode(plbRst, plbABus, plbPAValid, plbRNW, plbWrDBus, rdData, addrPref)
      | 
      | % constant variables (TODO: should pass from outside)
      | ADDRPREF_LEN = 16;
      | BANKADDR_LEN = 2;
      | LINEARADDR_LEN = 12;
      | ABUS_LEN = 32;
      | DBUS_LEN = 32;
      | 
      | % declare and initialize persistent variables
      | % register input bus signals
      | persistent plbRstReg_, plbRstReg_ = xl_state(0, {xlBoolean});
      | persistent plbABusReg_, plbABusReg_ = xl_state(0, {xlUnsigned, ABUS_LEN, 0});
      | persistent plbPAValidReg_, plbPAValidReg_ = xl_state(0, {xlBoolean});
      | persistent plbRNWReg_, plbRNWReg_ = xl_state(0, {xlUnsigned, 1, 0});
      | persistent plbWrDBusReg_, plbWrDBusReg_ = xl_state(0, {xlUnsigned, DBUS_LEN, 0});
      | 
      | % ===== rest of the outputs =====
      | 
      | bankAddr   = xl_slice(plbABusReg_, 2+BANKADDR_LEN+LINEARADDR_LEN-1, 2+LINEARADDR_LEN);
      | linearAddr = xl_slice(plbABusReg_, 2+LINEARADDR_LEN-1, 2);
      | RNWReg = plbRNWReg_;
      | wrDBusReg = plbWrDBusReg_;
      | 
      | % ===== p_select =====
      | 
      | % register PAValid
      | persistent aValidReg, aValidReg = xl_state(0, {xlBoolean});
      | aValidReg = plbPAValidReg_;
      | 
      | % extract and register the address prefix
      | addrPref_in = xl_slice(plbABusReg_, xl_nbits(plbABusReg_)-1, xl_nbits(plbABusReg_)-ADDRPREF_LEN);
      | if addrPref_in == addrPref
      |     ps1 = true;
      | else 
      |     ps1 = false;
      | end 
      | 
      | persistent ps1Reg, ps1Reg = xl_state(0, ps1);
      | ps1Reg = ps1;
      | 
      | ps = xl_and(ps1Reg, aValidReg);
      | 
      | % ===== addrAck =====
      | 
      | % register ps
      | persistent psReg, psReg = xl_state(0, ps);
      | 
      | addrAck = xfix({xlUnsigned, 1, 0}, xl_and(xl_not(plbRstReg_), ps, xl_not(psReg)));
      | 
      | psReg = ps;
      | 
      | % ===== rdComp, rd/wr DAck =====
      |  
      | rdComp1 = xfix({xlUnsigned, 1, 0}, xl_and(addrAck, RNWReg));
      | 
      | NUM_rdCompDelay = 3;
      | persistent rdCompDelay, rdCompDelay = xl_state(zeros(1, NUM_rdCompDelay), rdComp1, NUM_rdCompDelay);
      | rdComp2 = rdCompDelay.back;
      | rdCompDelay.push_front_pop_back(rdComp1);
      | 
      | persistent rdCompReg, rdCompReg = xl_state(0, rdComp1);
      | rdComp = rdCompReg;
      | rdCompReg = rdComp2;
      | 
      | persistent rdDAckReg, rdDAckReg = xl_state(0, rdComp1);
      | rdDAck = rdDAckReg;
      | rdDAckReg = rdComp;
      | 
      | persistent wrDAckReg, wrDAckReg = xl_state(0, addrAck);
      | wrDAck = wrDAckReg;
      | wrDAckReg = xl_and(addrAck, xl_not(RNWReg));
      | 
      | % ===== rdDBus =====
      | 
      | rdSel = xl_or(rdComp2, rdComp);
      | 
      | if rdSel == 1
      |     rdDBus1 = rdData;
      | else
      |     rdDBus1 = 0;
      | end % if
      | 
      | persistent rdDBusReg, rdDBusReg = xl_state(0, rdDBus1);
      | rdDBus = rdDBusReg;
      | rdDBusReg = rdDBus1;
      | 
      | % rdDBus = xl_concat(rdDBus32, rdDBus32);
      | % rdDBus = rdDBus32;
      | 
      | % ===== update the persistent variables =====
      | 
      | plbRstReg_ = plbRst;
      | plbABusReg_ = plbABus;
      | plbPAValidReg_ = plbPAValid;
      | plbRNWReg_ = plbRNW;
      | plbWrDBusReg_ = xl_slice(plbWrDBus, DBUS_LEN-1, 0);
  plb_memmap : xbsIndex_r4/MCode  mfname=xlmax  explicit_period=off  period=1  dbl_ovrd=off  enable_stdout=off  enable_debug=off  mfilecontent=|  suppress_output=on  defparams={}  hide_port_list={}  block_type=mcode
      | function [read_bank_out, sm_FEC_Config_din, sm_FEC_Config_en, sm_Tx_OFDM_SymCounts_din, sm_Tx_OFDM_SymCounts_en, sm_Rx_OFDM_SymbolCounts_din, sm_Rx_OFDM_SymbolCounts_en, sm_TxRx_Pilots_Values_din, sm_TxRx_Pilots_Values_en, sm_TxRx_Pilots_Index_din, sm_TxRx_Pilots_Index_en, sm_TxRx_Interrupt_PktBuf_Ctrl_din, sm_TxRx_Interrupt_PktBuf_Ctrl_en, sm_TxRx_FFT_Scaling_din, sm_TxRx_FFT_Scaling_en, sm_Tx_Start_Reset_Control_din, sm_Tx_Start_Reset_Control_en, sm_Tx_ControlBits_din, sm_Tx_ControlBits_en, sm_Tx_Delays_din, sm_Tx_Delays_en, sm_Tx_Scaling_din, sm_Tx_Scaling_en, sm_pktDet_thresholds_din, sm_pktDet_thresholds_en, sm_pktDet_durations_din, sm_pktDet_durations_en, sm_pktDet_autoCorrParams_din, sm_pktDet_autoCorrParams_en, sm_pktDet_controlBits_din, sm_pktDet_controlBits_en, sm_Rx_AF_TxScaling_din, sm_Rx_AF_TxScaling_en, sm_Rx_AF_Blanking_din, sm_Rx_AF_Blanking_en, sm_Rx_PilotCalcParams_din, sm_Rx_PilotCalcParams_en, sm_Rx_Constellation_Scaling_din, sm_Rx_Constellation_Scaling_en, sm_Rx_PktDet_Delay_din, sm_Rx_PktDet_Delay_en, sm_Rx_ChanEst_MinMag_din, sm_Rx_ChanEst_MinMag_en, sm_Rx_pktByteNums_din, sm_Rx_pktByteNums_en, sm_Rx_FixedPktLen_din, sm_Rx_FixedPktLen_en, sm_Rx_PktDet_LongCorr_Thresholds_din, sm_Rx_PktDet_LongCorr_Thresholds_en, sm_Rx_PktDet_LongCorr_Params_din, sm_Rx_PktDet_LongCorr_Params_en, sm_Rx_coarseCFO_correction_din, sm_Rx_coarseCFO_correction_en, sm_Rx_PreCFO_PilotCalcCorrection_din, sm_Rx_PreCFO_PilotCalcCorrection_en, sm_Rx_PreCFO_Options_din, sm_Rx_PreCFO_Options_en, sm_Rx_ControlBits_din, sm_Rx_ControlBits_en, sm_TxRx_AutoReply_Action3_din, sm_TxRx_AutoReply_Action3_en, sm_TxRx_AutoReply_Action2_din, sm_TxRx_AutoReply_Action2_en, sm_TxRx_AutoReply_Action1_din, sm_TxRx_AutoReply_Action1_en, sm_TxRx_AutoReply_Action0_din, sm_TxRx_AutoReply_Action0_en, sm_TxRx_AutoReply_Match5_din, sm_TxRx_AutoReply_Match5_en, sm_TxRx_AutoReply_Match4_din, sm_TxRx_AutoReply_Match4_en, sm_TxRx_AutoReply_Match3_din, sm_TxRx_AutoReply_Match3_en, sm_TxRx_AutoReply_Match2_din, sm_TxRx_AutoReply_Match2_en, sm_TxRx_AutoReply_Action5_din, sm_TxRx_AutoReply_Action5_en, sm_TxRx_AutoReply_Action4_din, sm_TxRx_AutoReply_Action4_en, sm_TxRx_AutoReply_Match1_din, sm_TxRx_AutoReply_Match1_en, sm_TxRx_AutoReply_Match0_din, sm_TxRx_AutoReply_Match0_en, sm_TxModulation_addr, sm_TxModulation_din, sm_TxModulation_we, sm_TxHeaderTranslate_addr, sm_TxHeaderTranslate_din, sm_TxHeaderTranslate_we, sm_ChannelEstimates_addr, sm_ChannelEstimates_din, sm_ChannelEstimates_we, sm_RxModulation_addr, sm_RxModulation_din, sm_RxModulation_we, sm_EVM_perSym_addr, sm_EVM_perSym_din, sm_EVM_perSym_we, sm_EVM_perSC_addr, sm_EVM_perSC_din, sm_EVM_perSC_we, sm_PktBufFreqOffsets_addr, sm_PktBufFreqOffsets_din, sm_PktBufFreqOffsets_we] = plb_memmap(wrDBus, bankAddr, linearAddr, RNWReg, addrAck, sm_midPacketRSSI, sm_Tx_PktRunning, sm_Rx_pktDetEventCount, sm_Rx_coarseCFOest, sm_Rx_pilotCFOest, sm_Rx_pktDone_interruptStatus, sm_Rx_BER_TotalBits, sm_Rx_BER_Errors, sm_Rx_Gains, sm_pktDet_status, sm_FEC_Config, sm_Tx_OFDM_SymCounts, sm_Rx_OFDM_SymbolCounts, sm_TxRx_Pilots_Values, sm_TxRx_Pilots_Index, sm_TxRx_Interrupt_PktBuf_Ctrl, sm_TxRx_FFT_Scaling, sm_Tx_Start_Reset_Control, sm_Tx_ControlBits, sm_Tx_Delays, sm_Tx_Scaling, sm_pktDet_thresholds, sm_pktDet_durations, sm_pktDet_autoCorrParams, sm_pktDet_controlBits, sm_Rx_AF_TxScaling, sm_Rx_AF_Blanking, sm_Rx_PilotCalcParams, sm_Rx_Constellation_Scaling, sm_Rx_PktDet_Delay, sm_Rx_ChanEst_MinMag, sm_Rx_pktByteNums, sm_Rx_FixedPktLen, sm_Rx_PktDet_LongCorr_Thresholds, sm_Rx_PktDet_LongCorr_Params, sm_Rx_coarseCFO_correction, sm_Rx_PreCFO_PilotCalcCorrection, sm_Rx_PreCFO_Options, sm_Rx_ControlBits, sm_TxRx_AutoReply_Action3, sm_TxRx_AutoReply_Action2, sm_TxRx_AutoReply_Action1, sm_TxRx_AutoReply_Action0, sm_TxRx_AutoReply_Match5, sm_TxRx_AutoReply_Match4, sm_TxRx_AutoReply_Match3, sm_TxRx_AutoReply_Match2, sm_TxRx_AutoReply_Action5, sm_TxRx_AutoReply_Action4, sm_TxRx_AutoReply_Match1, sm_TxRx_AutoReply_Match0, sm_TxModulation, sm_TxHeaderTranslate, sm_ChannelEstimates, sm_RxModulation, sm_EVM_perSym, sm_EVM_perSC, sm_PktBufFreqOffsets)
      | 
      | 
      | % connvert the input data to UFix_32_0 (the bus data type)
      | % 'From Register' blocks
      | % sm_midPacketRSSI_bus = xfix({xlUnsigned, 32, 0}, 0);
      | sm_midPacketRSSI_bus = xl_force(sm_midPacketRSSI, xlUnsigned, 0);
      | 
      | % sm_Tx_PktRunning_bus = xfix({xlUnsigned, 32, 0}, 0);
      | sm_Tx_PktRunning_bus = xl_force(sm_Tx_PktRunning, xlUnsigned, 0);
      | 
      | % sm_Rx_pktDetEventCount_bus = xfix({xlUnsigned, 32, 0}, 0);
      | sm_Rx_pktDetEventCount_bus = xl_force(sm_Rx_pktDetEventCount, xlUnsigned, 0);
      | 
      | % sm_Rx_coarseCFOest_bus = xfix({xlUnsigned, 32, 0}, 0);
      | sm_Rx_coarseCFOest_bus = xl_force(sm_Rx_coarseCFOest, xlUnsigned, 0);
      | 
      | % sm_Rx_pilotCFOest_bus = xfix({xlUnsigned, 32, 0}, 0);
      | sm_Rx_pilotCFOest_bus = xl_force(sm_Rx_pilotCFOest, xlUnsigned, 0);
      | 
      | % sm_Rx_pktDone_interruptStatus_bus = xfix({xlUnsigned, 32, 0}, 0);
      | sm_Rx_pktDone_interruptStatus_bus = xl_force(sm_Rx_pktDone_interruptStatus, xlUnsigned, 0);
      | 
      | % sm_Rx_BER_TotalBits_bus = xfix({xlUnsigned, 32, 0}, 0);
      | sm_Rx_BER_TotalBits_bus = xl_force(sm_Rx_BER_TotalBits, xlUnsigned, 0);
      | 
      | % sm_Rx_BER_Errors_bus = xfix({xlUnsigned, 32, 0}, 0);
      | sm_Rx_BER_Errors_bus = xl_force(sm_Rx_BER_Errors, xlUnsigned, 0);
      | 
      | % sm_Rx_Gains_bus = xfix({xlUnsigned, 32, 0}, 0);
      | sm_Rx_Gains_bus = xl_force(sm_Rx_Gains, xlUnsigned, 0);
      | 
      | % sm_pktDet_status_bus = xfix({xlUnsigned, 32, 0}, 0);
      | sm_pktDet_status_bus = xl_force(sm_pktDet_status, xlUnsigned, 0);
      | 
      | % 'To Register' blocks
      | 
      | % sm_FEC_Config_dout = xfix({xlUnsigned, 32, 0}, 0);
      | sm_FEC_Config_dout = xl_force(sm_FEC_Config, xlUnsigned, 0);
      | 
      | % sm_Tx_OFDM_SymCounts_dout = xfix({xlUnsigned, 32, 0}, 0);
      | sm_Tx_OFDM_SymCounts_dout = xl_force(sm_Tx_OFDM_SymCounts, xlUnsigned, 0);
      | 
      | % sm_Rx_OFDM_SymbolCounts_dout = xfix({xlUnsigned, 32, 0}, 0);
      | sm_Rx_OFDM_SymbolCounts_dout = xl_force(sm_Rx_OFDM_SymbolCounts, xlUnsigned, 0);
      | 
      | % sm_TxRx_Pilots_Values_dout = xfix({xlUnsigned, 32, 0}, 0);
      | sm_TxRx_Pilots_Values_dout = xl_force(sm_TxRx_Pilots_Values, xlUnsigned, 0);
      | 
      | % sm_TxRx_Pilots_Index_dout = xfix({xlUnsigned, 32, 0}, 0);
      | sm_TxRx_Pilots_Index_dout = xl_force(sm_TxRx_Pilots_Index, xlUnsigned, 0);
      | 
      | % sm_TxRx_Interrupt_PktBuf_Ctrl_dout = xfix({xlUnsigned, 32, 0}, 0);
      | sm_TxRx_Interrupt_PktBuf_Ctrl_dout = xl_force(sm_TxRx_Interrupt_PktBuf_Ctrl, xlUnsigned, 0);
      | 
      | % sm_TxRx_FFT_Scaling_dout = xfix({xlUnsigned, 32, 0}, 0);
      | sm_TxRx_FFT_Scaling_dout = xl_force(sm_TxRx_FFT_Scaling, xlUnsigned, 0);
      | 
      | % sm_Tx_Start_Reset_Control_dout = xfix({xlUnsigned, 32, 0}, 0);
      | sm_Tx_Start_Reset_Control_dout = xl_force(sm_Tx_Start_Reset_Control, xlUnsigned, 0);
      | 
      | % sm_Tx_ControlBits_dout = xfix({xlUnsigned, 32, 0}, 0);
      | sm_Tx_ControlBits_dout = xl_force(sm_Tx_ControlBits, xlUnsigned, 0);
      | 
      | % sm_Tx_Delays_dout = xfix({xlUnsigned, 32, 0}, 0);
      | sm_Tx_Delays_dout = xl_force(sm_Tx_Delays, xlUnsigned, 0);
      | 
      | % sm_Tx_Scaling_dout = xfix({xlUnsigned, 32, 0}, 0);
      | sm_Tx_Scaling_dout = xl_force(sm_Tx_Scaling, xlUnsigned, 0);
      | 
      | % sm_pktDet_thresholds_dout = xfix({xlUnsigned, 32, 0}, 0);
      | sm_pktDet_thresholds_dout = xl_force(sm_pktDet_thresholds, xlUnsigned, 0);
      | 
      | % sm_pktDet_durations_dout = xfix({xlUnsigned, 32, 0}, 0);
      | sm_pktDet_durations_dout = xl_force(sm_pktDet_durations, xlUnsigned, 0);
      | 
      | % sm_pktDet_autoCorrParams_dout = xfix({xlUnsigned, 32, 0}, 0);
      | sm_pktDet_autoCorrParams_dout = xl_force(sm_pktDet_autoCorrParams, xlUnsigned, 0);
      | 
      | % sm_pktDet_controlBits_dout = xfix({xlUnsigned, 32, 0}, 0);
      | sm_pktDet_controlBits_dout = xl_force(sm_pktDet_controlBits, xlUnsigned, 0);
      | 
      | % sm_Rx_AF_TxScaling_dout = xfix({xlUnsigned, 32, 0}, 0);
      | sm_Rx_AF_TxScaling_dout = xl_force(sm_Rx_AF_TxScaling, xlUnsigned, 0);
      | 
      | % sm_Rx_AF_Blanking_dout = xfix({xlUnsigned, 32, 0}, 0);
      | sm_Rx_AF_Blanking_dout = xl_force(sm_Rx_AF_Blanking, xlUnsigned, 0);
      | 
      | % sm_Rx_PilotCalcParams_dout = xfix({xlUnsigned, 32, 0}, 0);
      | sm_Rx_PilotCalcParams_dout = xl_force(sm_Rx_PilotCalcParams, xlUnsigned, 0);
      | 
      | % sm_Rx_Constellation_Scaling_dout = xfix({xlUnsigned, 32, 0}, 0);
      | sm_Rx_Constellation_Scaling_dout = xl_force(sm_Rx_Constellation_Scaling, xlUnsigned, 0);
      | 
      | % sm_Rx_PktDet_Delay_dout = xfix({xlUnsigned, 32, 0}, 0);
      | sm_Rx_PktDet_Delay_dout = xl_force(sm_Rx_PktDet_Delay, xlUnsigned, 0);
      | 
      | % sm_Rx_ChanEst_MinMag_dout = xfix({xlUnsigned, 32, 0}, 0);
      | sm_Rx_ChanEst_MinMag_dout = xl_force(sm_Rx_ChanEst_MinMag, xlUnsigned, 0);
      | 
      | % sm_Rx_pktByteNums_dout = xfix({xlUnsigned, 32, 0}, 0);
      | sm_Rx_pktByteNums_dout = xl_force(sm_Rx_pktByteNums, xlUnsigned, 0);
      | 
      | % sm_Rx_FixedPktLen_dout = xfix({xlUnsigned, 32, 0}, 0);
      | sm_Rx_FixedPktLen_dout = xl_force(sm_Rx_FixedPktLen, xlUnsigned, 0);
      | 
      | % sm_Rx_PktDet_LongCorr_Thresholds_dout = xfix({xlUnsigned, 32, 0}, 0);
      | sm_Rx_PktDet_LongCorr_Thresholds_dout = xl_force(sm_Rx_PktDet_LongCorr_Thresholds, xlUnsigned, 0);
      | 
      | % sm_Rx_PktDet_LongCorr_Params_dout = xfix({xlUnsigned, 32, 0}, 0);
      | sm_Rx_PktDet_LongCorr_Params_dout = xl_force(sm_Rx_PktDet_LongCorr_Params, xlUnsigned, 0);
      | 
      | % sm_Rx_coarseCFO_correction_dout = xfix({xlUnsigned, 32, 0}, 0);
      | sm_Rx_coarseCFO_correction_dout = xl_force(sm_Rx_coarseCFO_correction, xlUnsigned, 0);
      | 
      | % sm_Rx_PreCFO_PilotCalcCorrection_dout = xfix({xlUnsigned, 32, 0}, 0);
      | sm_Rx_PreCFO_PilotCalcCorrection_dout = xl_force(sm_Rx_PreCFO_PilotCalcCorrection, xlUnsigned, 0);
      | 
      | % sm_Rx_PreCFO_Options_dout = xfix({xlUnsigned, 32, 0}, 0);
      | sm_Rx_PreCFO_Options_dout = xl_force(sm_Rx_PreCFO_Options, xlUnsigned, 0);
      | 
      | % sm_Rx_ControlBits_dout = xfix({xlUnsigned, 32, 0}, 0);
      | sm_Rx_ControlBits_dout = xl_force(sm_Rx_ControlBits, xlUnsigned, 0);
      | 
      | % sm_TxRx_AutoReply_Action3_dout = xfix({xlUnsigned, 32, 0}, 0);
      | sm_TxRx_AutoReply_Action3_dout = xl_force(sm_TxRx_AutoReply_Action3, xlUnsigned, 0);
      | 
      | % sm_TxRx_AutoReply_Action2_dout = xfix({xlUnsigned, 32, 0}, 0);
      | sm_TxRx_AutoReply_Action2_dout = xl_force(sm_TxRx_AutoReply_Action2, xlUnsigned, 0);
      | 
      | % sm_TxRx_AutoReply_Action1_dout = xfix({xlUnsigned, 32, 0}, 0);
      | sm_TxRx_AutoReply_Action1_dout = xl_force(sm_TxRx_AutoReply_Action1, xlUnsigned, 0);
      | 
      | % sm_TxRx_AutoReply_Action0_dout = xfix({xlUnsigned, 32, 0}, 0);
      | sm_TxRx_AutoReply_Action0_dout = xl_force(sm_TxRx_AutoReply_Action0, xlUnsigned, 0);
      | 
      | % sm_TxRx_AutoReply_Match5_dout = xfix({xlUnsigned, 32, 0}, 0);
      | sm_TxRx_AutoReply_Match5_dout = xl_force(sm_TxRx_AutoReply_Match5, xlUnsigned, 0);
      | 
      | % sm_TxRx_AutoReply_Match4_dout = xfix({xlUnsigned, 32, 0}, 0);
      | sm_TxRx_AutoReply_Match4_dout = xl_force(sm_TxRx_AutoReply_Match4, xlUnsigned, 0);
      | 
      | % sm_TxRx_AutoReply_Match3_dout = xfix({xlUnsigned, 32, 0}, 0);